simulink model "os_polyphase_synth_4096c"  (R2021a) (continued)
== system os_polyphase_synth_4096c/pfb_fir/pol1_in1_tap26 == (continued)
      | P+C+CONCAT+PCIN
      | P+C+CONCAT+PCIN+CARRYIN
      | P+C+CONCAT+PCIN>>17
      | P+C+CONCAT+PCIN>>17+CARRYIN
      | P+C+CONCAT-C
      | P+C+CONCAT-P
      | P+C+CONCAT-P>>17
      | P+C+CONCAT-PCIN
      | P+C+CONCAT-PCIN>>17
      | P+C+D
      | P+C+D*B
      | P+C+D*B+CARRYIN
      | P+C+D*BCIN
      | P+C+D*BCIN+CARRYIN
      | P+C+D*D
      | P+C+D*D+CARRYIN
      | P+C+D+CARRYIN
      | P+C+P
      | P+C+P+C
      | P+C+P+C+CARRYIN
      | P+C+P+CARRYIN
      | P+C+P+CONCAT
      | P+C+P+CONCAT+CARRYIN
      | P+C+P+P
      | P+C+P+P+CARRYIN
      | P+C+P+P>>17
      | P+C+P+P>>17+CARRYIN
      | P+C+P+PCIN
      | P+C+P+PCIN+CARRYIN
      | P+C+P+PCIN>>17
      | P+C+P+PCIN>>17+CARRYIN
      | P+C+P-C
      | P+C+P-P
      | P+C+P-P>>17
      | P+C+P-PCIN
      | P+C+P-PCIN>>17
      | P+C+P>>17
      | P+C+P>>17+C
      | P+C+P>>17+C+CARRYIN
      | P+C+P>>17+CARRYIN
      | P+C+P>>17+CONCAT
      | P+C+P>>17+CONCAT+CARRYIN
      | P+C+P>>17+P
      | P+C+P>>17+P+CARRYIN
      | P+C+PCIN
      | P+C+PCIN+C
      | P+C+PCIN+C+CARRYIN
      | P+C+PCIN+CARRYIN
      | P+C+PCIN+CONCAT
      | P+C+PCIN+CONCAT+CARRYIN
      | P+C+PCIN+P
      | P+C+PCIN+P+CARRYIN
      | P+C+PCIN>>17
      | P+C+PCIN>>17+C
      | P+C+PCIN>>17+C+CARRYIN
      | P+C+PCIN>>17+CARRYIN
      | P+C+PCIN>>17+CONCAT
      | P+C+PCIN>>17+CONCAT+CARRYIN
      | P+C+PCIN>>17+P
      | P+C+PCIN>>17+P+CARRYIN
      | P+C-C
      | P+C-P
      | P+C-P>>17
      | P+C-PCIN
      | P+C-PCIN>>17
      | P+CARRYCASCIN
      | P+CARRYIN
      | P+CONCAT
      | P+CONCAT+C
      | P+CONCAT+C+C
      | P+CONCAT+C+C+CARRYIN
      | P+CONCAT+C+CARRYIN
      | P+CONCAT+C+P
      | P+CONCAT+C+P+CARRYIN
      | P+CONCAT+C+P>>17
      | P+CONCAT+C+P>>17+CARRYIN
      | P+CONCAT+C+PCIN
      | P+CONCAT+C+PCIN+CARRYIN
      | P+CONCAT+C+PCIN>>17
      | P+CONCAT+C+PCIN>>17+CARRYIN
      | P+CONCAT+C-C
      | P+CONCAT+C-P
      | P+CONCAT+C-P>>17
      | P+CONCAT+C-PCIN
      | P+CONCAT+C-PCIN>>17
      | P+CONCAT+CARRYCASCIN
      | P+CONCAT+CARRYIN
      | P+CONCAT+P
      | P+CONCAT+P+C
      | P+CONCAT+P+C+CARRYIN
      | P+CONCAT+P+CARRYIN
      | P+CONCAT+P>>17
      | P+CONCAT+P>>17+C
      | P+CONCAT+P>>17+C+CARRYIN
      | P+CONCAT+P>>17+CARRYIN
      | P+CONCAT+PCIN
      | P+CONCAT+PCIN+C
      | P+CONCAT+PCIN+C+CARRYIN
      | P+CONCAT+PCIN+CARRYIN
      | P+CONCAT+PCIN>>17
      | P+CONCAT+PCIN>>17+C
      | P+CONCAT+PCIN>>17+C+CARRYIN
      | P+CONCAT+PCIN>>17+CARRYIN
      | P+CONCAT-C
      | P+CONCAT-P
      | P+CONCAT-P>>17
      | P+CONCAT-PCIN
      | P+CONCAT-PCIN>>17
      | P+D
      | P+D*B
      | P+D*B+C
      | P+D*B+C+CARRYIN
      | P+D*B+CARRYIN
      | P+D*B+P
      | P+D*B+P+CARRYIN
      | P+D*B+P>>17
      | P+D*B+P>>17+CARRYIN
      | P+D*B+PCIN
      | P+D*B+PCIN+CARRYIN
      | P+D*B+PCIN>>17
      | P+D*B+PCIN>>17+CARRYIN
      | P+D*B-C
      | P+D*B-P
      | P+D*B-P>>17
      | P+D*B-PCIN
      | P+D*B-PCIN>>17
      | P+D*BCIN
      | P+D*BCIN+C
      | P+D*BCIN+C+CARRYIN
      | P+D*BCIN+CARRYIN
      | P+D*BCIN+P
      | P+D*BCIN+P+CARRYIN
      | P+D*BCIN+P>>17
      | P+D*BCIN+P>>17+CARRYIN
      | P+D*BCIN+PCIN
      | P+D*BCIN+PCIN+CARRYIN
      | P+D*BCIN+PCIN>>17
      | P+D*BCIN+PCIN>>17+CARRYIN
      | P+D*BCIN-C
      | P+D*BCIN-P
      | P+D*BCIN-P>>17
      | P+D*BCIN-PCIN
      | P+D*BCIN-PCIN>>17
      | P+D*D
      | P+D*D+C
      | P+D*D+C+CARRYIN
      | P+D*D+CARRYIN
      | P+D*D+P
      | P+D*D+P+CARRYIN
      | P+D*D+P>>17
      | P+D*D+P>>17+CARRYIN
      | P+D*D+PCIN
      | P+D*D+PCIN+CARRYIN
      | P+D*D+PCIN>>17
      | P+D*D+PCIN>>17+CARRYIN
      | P+D*D-C
      | P+D*D-P
      | P+D*D-P>>17
      | P+D*D-PCIN
      | P+D*D-PCIN>>17
      | P+D+C
      | P+D+C+CARRYIN
      | P+D+CARRYIN
      | P+D+P
      | P+D+P+CARRYIN
      | P+D+P>>17
      | P+D+P>>17+CARRYIN
      | P+D+PCIN
      | P+D+PCIN+CARRYIN
      | P+D+PCIN>>17
      | P+D+PCIN>>17+CARRYIN
      | P+D-C
      | P+D-P
      | P+D-P>>17
      | P+D-PCIN
      | P+D-PCIN>>17
      | P+P
      | P+P+(A+D)
      | P+P+(A+D)*(A+D)
      | P+P+(A+D)*(A+D)+CARRYIN
      | P+P+(A+D)*B
      | P+P+(A+D)*B+CARRYIN
      | P+P+(A+D)*BCIN
      | P+P+(A+D)*BCIN+CARRYIN
      | P+P+(A+D)+CARRYIN
      | P+P+(ACIN+D)
      | P+P+(ACIN+D)*(ACIN+D)
      | P+P+(ACIN+D)*(ACIN+D)+CARRYIN
      | P+P+(ACIN+D)*B
      | P+P+(ACIN+D)*B+CARRYIN
      | P+P+(ACIN+D)*BCIN
      | P+P+(ACIN+D)*BCIN+CARRYIN
      | P+P+(ACIN+D)+CARRYIN
      | P+P+(D+A)
      | P+P+(D+A)*(D+A)
      | P+P+(D+A)*(D+A)+CARRYIN
      | P+P+(D+A)*B
      | P+P+(D+A)*B+CARRYIN
      | P+P+(D+A)*BCIN
      | P+P+(D+A)*BCIN+CARRYIN
      | P+P+(D+A)+CARRYIN
      | P+P+(D+ACIN)
      | P+P+(D+ACIN)*(D+ACIN)
      | P+P+(D+ACIN)*(D+ACIN)+CARRYIN
      | P+P+(D+ACIN)*B
      | P+P+(D+ACIN)*B+CARRYIN
      | P+P+(D+ACIN)*BCIN
      | P+P+(D+ACIN)*BCIN+CARRYIN
      | P+P+(D+ACIN)+CARRYIN
      | P+P+(D-A)
      | P+P+(D-A)*(D-A)
      | P+P+(D-A)*(D-A)+CARRYIN
      | P+P+(D-A)*B
      | P+P+(D-A)*B+CARRYIN
      | P+P+(D-A)*BCIN
      | P+P+(D-A)*BCIN+CARRYIN
      | P+P+(D-A)+CARRYIN
      | P+P+(D-ACIN)
      | P+P+(D-ACIN)*(D-ACIN)
      | P+P+(D-ACIN)*(D-ACIN)+CARRYIN
      | P+P+(D-ACIN)*B
      | P+P+(D-ACIN)*B+CARRYIN
      | P+P+(D-ACIN)*BCIN
      | P+P+(D-ACIN)*BCIN+CARRYIN
      | P+P+(D-ACIN)+CARRYIN
      | P+P+A
      | P+P+A*A
      | P+P+A*A+CARRYIN
      | P+P+A*B
      | P+P+A*B+CARRYIN
      | P+P+A*BCIN
      | P+P+A*BCIN+CARRYIN
      | P+P+A+CARRYIN
      | P+P+ACIN
      | P+P+ACIN*ACIN
      | P+P+ACIN*ACIN+CARRYIN
      | P+P+ACIN*B
      | P+P+ACIN*B+CARRYIN
      | P+P+ACIN*BCIN
      | P+P+ACIN*BCIN+CARRYIN
      | P+P+ACIN+CARRYIN
      | P+P+B*(A+D)
      | P+P+B*(A+D)+CARRYIN
      | P+P+B*(ACIN+D)
      | P+P+B*(ACIN+D)+CARRYIN
      | P+P+B*(D+A)
      | P+P+B*(D+A)+CARRYIN
      | P+P+B*(D+ACIN)
      | P+P+B*(D+ACIN)+CARRYIN
      | P+P+B*(D-A)
      | P+P+B*(D-A)+CARRYIN
      | P+P+B*(D-ACIN)
      | P+P+B*(D-ACIN)+CARRYIN
      | P+P+B*A
      | P+P+B*A+CARRYIN
      | P+P+B*ACIN
      | P+P+B*ACIN+CARRYIN
      | P+P+B*D
      | P+P+B*D+CARRYIN
      | P+P+BCIN*(A+D)
      | P+P+BCIN*(A+D)+CARRYIN
      | P+P+BCIN*(ACIN+D)
      | P+P+BCIN*(ACIN+D)+CARRYIN
      | P+P+BCIN*(D+A)
      | P+P+BCIN*(D+A)+CARRYIN
      | P+P+BCIN*(D+ACIN)
      | P+P+BCIN*(D+ACIN)+CARRYIN
      | P+P+BCIN*(D-A)
      | P+P+BCIN*(D-A)+CARRYIN
      | P+P+BCIN*(D-ACIN)
      | P+P+BCIN*(D-ACIN)+CARRYIN
      | P+P+BCIN*A
      | P+P+BCIN*A+CARRYIN
      | P+P+BCIN*ACIN
      | P+P+BCIN*ACIN+CARRYIN
      | P+P+BCIN*D
      | P+P+BCIN*D+CARRYIN
      | P+P+C
      | P+P+C+C
      | P+P+C+C+CARRYIN
      | P+P+C+CARRYIN
      | P+P+C+CONCAT
      | P+P+C+CONCAT+CARRYIN
      | P+P+C+P
      | P+P+C+P+CARRYIN
      | P+P+C+P>>17
      | P+P+C+P>>17+CARRYIN
      | P+P+C+PCIN
      | P+P+C+PCIN+CARRYIN
      | P+P+C+PCIN>>17
      | P+P+C+PCIN>>17+CARRYIN
      | P+P+C-C
      | P+P+C-P
      | P+P+C-P>>17
      | P+P+C-PCIN
      | P+P+C-PCIN>>17
      | P+P+CARRYCASCIN
      | P+P+CARRYIN
      | P+P+CONCAT
      | P+P+CONCAT+C
      | P+P+CONCAT+C+CARRYIN
      | P+P+CONCAT+CARRYIN
      | P+P+D
      | P+P+D*B
      | P+P+D*B+CARRYIN
      | P+P+D*BCIN
      | P+P+D*BCIN+CARRYIN
      | P+P+D*D
      | P+P+D*D+CARRYIN
      | P+P+D+CARRYIN
      | P+P+P
      | P+P+P+C
      | P+P+P+C+CARRYIN
      | P+P+P+CARRYIN
      | P+P+P>>17
      | P+P+P>>17+C
      | P+P+P>>17+C+CARRYIN
      | P+P+P>>17+CARRYIN
      | P+P+PCIN
      | P+P+PCIN+C
      | P+P+PCIN+C+CARRYIN
      | P+P+PCIN+CARRYIN
      | P+P+PCIN>>17
      | P+P+PCIN>>17+C
      | P+P+PCIN>>17+C+CARRYIN
      | P+P+PCIN>>17+CARRYIN
      | P+P-C
      | P+P-P
      | P+P-P>>17
      | P+P-PCIN
      | P+P-PCIN>>17
      | P+P>>17
      | P+P>>17+(A+D)
      | P+P>>17+(A+D)*(A+D)
      | P+P>>17+(A+D)*(A+D)+CARRYIN
      | P+P>>17+(A+D)*B
      | P+P>>17+(A+D)*B+CARRYIN
      | P+P>>17+(A+D)*BCIN
      | P+P>>17+(A+D)*BCIN+CARRYIN
      | P+P>>17+(A+D)+CARRYIN
      | P+P>>17+(ACIN+D)
      | P+P>>17+(ACIN+D)*(ACIN+D)
      | P+P>>17+(ACIN+D)*(ACIN+D)+CARRYIN
      | P+P>>17+(ACIN+D)*B
      | P+P>>17+(ACIN+D)*B+CARRYIN
      | P+P>>17+(ACIN+D)*BCIN
      | P+P>>17+(ACIN+D)*BCIN+CARRYIN
      | P+P>>17+(ACIN+D)+CARRYIN
      | P+P>>17+(D+A)
      | P+P>>17+(D+A)*(D+A)
      | P+P>>17+(D+A)*(D+A)+CARRYIN
      | P+P>>17+(D+A)*B
      | P+P>>17+(D+A)*B+CARRYIN
      | P+P>>17+(D+A)*BCIN
      | P+P>>17+(D+A)*BCIN+CARRYIN
      | P+P>>17+(D+A)+CARRYIN
      | P+P>>17+(D+ACIN)
      | P+P>>17+(D+ACIN)*(D+ACIN)
      | P+P>>17+(D+ACIN)*(D+ACIN)+CARRYIN
      | P+P>>17+(D+ACIN)*B
      | P+P>>17+(D+ACIN)*B+CARRYIN
      | P+P>>17+(D+ACIN)*BCIN
      | P+P>>17+(D+ACIN)*BCIN+CARRYIN
      | P+P>>17+(D+ACIN)+CARRYIN
      | P+P>>17+(D-A)
      | P+P>>17+(D-A)*(D-A)
      | P+P>>17+(D-A)*(D-A)+CARRYIN
      | P+P>>17+(D-A)*B
      | P+P>>17+(D-A)*B+CARRYIN
      | P+P>>17+(D-A)*BCIN
      | P+P>>17+(D-A)*BCIN+CARRYIN
      | P+P>>17+(D-A)+CARRYIN
      | P+P>>17+(D-ACIN)
      | P+P>>17+(D-ACIN)*(D-ACIN)
      | P+P>>17+(D-ACIN)*(D-ACIN)+CARRYIN
      | P+P>>17+(D-ACIN)*B
      | P+P>>17+(D-ACIN)*B+CARRYIN
      | P+P>>17+(D-ACIN)*BCIN
      | P+P>>17+(D-ACIN)*BCIN+CARRYIN
      | P+P>>17+(D-ACIN)+CARRYIN
      | P+P>>17+A
      | P+P>>17+A*A
      | P+P>>17+A*A+CARRYIN
      | P+P>>17+A*B
      | P+P>>17+A*B+CARRYIN
      | P+P>>17+A*BCIN
      | P+P>>17+A*BCIN+CARRYIN
      | P+P>>17+A+CARRYIN
      | P+P>>17+ACIN
      | P+P>>17+ACIN*ACIN
      | P+P>>17+ACIN*ACIN+CARRYIN
      | P+P>>17+ACIN*B
      | P+P>>17+ACIN*B+CARRYIN
      | P+P>>17+ACIN*BCIN
      | P+P>>17+ACIN*BCIN+CARRYIN
      | P+P>>17+ACIN+CARRYIN
      | P+P>>17+B*(A+D)
      | P+P>>17+B*(A+D)+CARRYIN
      | P+P>>17+B*(ACIN+D)
      | P+P>>17+B*(ACIN+D)+CARRYIN
      | P+P>>17+B*(D+A)
      | P+P>>17+B*(D+A)+CARRYIN
      | P+P>>17+B*(D+ACIN)
      | P+P>>17+B*(D+ACIN)+CARRYIN
      | P+P>>17+B*(D-A)
      | P+P>>17+B*(D-A)+CARRYIN
      | P+P>>17+B*(D-ACIN)
      | P+P>>17+B*(D-ACIN)+CARRYIN
      | P+P>>17+B*A
      | P+P>>17+B*A+CARRYIN
      | P+P>>17+B*ACIN
      | P+P>>17+B*ACIN+CARRYIN
      | P+P>>17+B*D
      | P+P>>17+B*D+CARRYIN
      | P+P>>17+BCIN*(A+D)
      | P+P>>17+BCIN*(A+D)+CARRYIN
      | P+P>>17+BCIN*(ACIN+D)
      | P+P>>17+BCIN*(ACIN+D)+CARRYIN
      | P+P>>17+BCIN*(D+A)
      | P+P>>17+BCIN*(D+A)+CARRYIN
      | P+P>>17+BCIN*(D+ACIN)
      | P+P>>17+BCIN*(D+ACIN)+CARRYIN
      | P+P>>17+BCIN*(D-A)
      | P+P>>17+BCIN*(D-A)+CARRYIN
      | P+P>>17+BCIN*(D-ACIN)
      | P+P>>17+BCIN*(D-ACIN)+CARRYIN
      | P+P>>17+BCIN*A
      | P+P>>17+BCIN*A+CARRYIN
      | P+P>>17+BCIN*ACIN
      | P+P>>17+BCIN*ACIN+CARRYIN
      | P+P>>17+BCIN*D
      | P+P>>17+BCIN*D+CARRYIN
      | P+P>>17+C
      | P+P>>17+C+C
      | P+P>>17+C+C+CARRYIN
      | P+P>>17+C+CARRYIN
      | P+P>>17+C+CONCAT
      | P+P>>17+C+CONCAT+CARRYIN
      | P+P>>17+C+P
      | P+P>>17+C+P+CARRYIN
      | P+P>>17+CARRYIN
      | P+P>>17+CONCAT
      | P+P>>17+CONCAT+C
      | P+P>>17+CONCAT+C+CARRYIN
      | P+P>>17+CONCAT+CARRYIN
      | P+P>>17+D
      | P+P>>17+D*B
      | P+P>>17+D*B+CARRYIN
      | P+P>>17+D*BCIN
      | P+P>>17+D*BCIN+CARRYIN
      | P+P>>17+D*D
      | P+P>>17+D*D+CARRYIN
      | P+P>>17+D+CARRYIN
      | P+P>>17+P
      | P+P>>17+P+C
      | P+P>>17+P+C+CARRYIN
      | P+P>>17+P+CARRYIN
      | P+PCIN
      | P+PCIN+(A+D)
      | P+PCIN+(A+D)*(A+D)
      | P+PCIN+(A+D)*(A+D)+CARRYIN
      | P+PCIN+(A+D)*B
      | P+PCIN+(A+D)*B+CARRYIN
      | P+PCIN+(A+D)*BCIN
      | P+PCIN+(A+D)*BCIN+CARRYIN
      | P+PCIN+(A+D)+CARRYIN
      | P+PCIN+(ACIN+D)
      | P+PCIN+(ACIN+D)*(ACIN+D)
      | P+PCIN+(ACIN+D)*(ACIN+D)+CARRYIN
      | P+PCIN+(ACIN+D)*B
      | P+PCIN+(ACIN+D)*B+CARRYIN
      | P+PCIN+(ACIN+D)*BCIN
      | P+PCIN+(ACIN+D)*BCIN+CARRYIN
      | P+PCIN+(ACIN+D)+CARRYIN
      | P+PCIN+(D+A)
      | P+PCIN+(D+A)*(D+A)
      | P+PCIN+(D+A)*(D+A)+CARRYIN
      | P+PCIN+(D+A)*B
      | P+PCIN+(D+A)*B+CARRYIN
      | P+PCIN+(D+A)*BCIN
      | P+PCIN+(D+A)*BCIN+CARRYIN
      | P+PCIN+(D+A)+CARRYIN
      | P+PCIN+(D+ACIN)
      | P+PCIN+(D+ACIN)*(D+ACIN)
      | P+PCIN+(D+ACIN)*(D+ACIN)+CARRYIN
      | P+PCIN+(D+ACIN)*B
      | P+PCIN+(D+ACIN)*B+CARRYIN
      | P+PCIN+(D+ACIN)*BCIN
      | P+PCIN+(D+ACIN)*BCIN+CARRYIN
      | P+PCIN+(D+ACIN)+CARRYIN
      | P+PCIN+(D-A)
      | P+PCIN+(D-A)*(D-A)
      | P+PCIN+(D-A)*(D-A)+CARRYIN
      | P+PCIN+(D-A)*B
      | P+PCIN+(D-A)*B+CARRYIN
      | P+PCIN+(D-A)*BCIN
      | P+PCIN+(D-A)*BCIN+CARRYIN
      | P+PCIN+(D-A)+CARRYIN
      | P+PCIN+(D-ACIN)
      | P+PCIN+(D-ACIN)*(D-ACIN)
      | P+PCIN+(D-ACIN)*(D-ACIN)+CARRYIN
      | P+PCIN+(D-ACIN)*B
      | P+PCIN+(D-ACIN)*B+CARRYIN
      | P+PCIN+(D-ACIN)*BCIN
      | P+PCIN+(D-ACIN)*BCIN+CARRYIN
      | P+PCIN+(D-ACIN)+CARRYIN
      | P+PCIN+A
      | P+PCIN+A*A
      | P+PCIN+A*A+CARRYIN
      | P+PCIN+A*B
      | P+PCIN+A*B+CARRYIN
      | P+PCIN+A*BCIN
      | P+PCIN+A*BCIN+CARRYIN
      | P+PCIN+A+CARRYIN
      | P+PCIN+ACIN
      | P+PCIN+ACIN*ACIN
      | P+PCIN+ACIN*ACIN+CARRYIN
      | P+PCIN+ACIN*B
      | P+PCIN+ACIN*B+CARRYIN
      | P+PCIN+ACIN*BCIN
      | P+PCIN+ACIN*BCIN+CARRYIN
      | P+PCIN+ACIN+CARRYIN
      | P+PCIN+B*(A+D)
      | P+PCIN+B*(A+D)+CARRYIN
      | P+PCIN+B*(ACIN+D)
      | P+PCIN+B*(ACIN+D)+CARRYIN
      | P+PCIN+B*(D+A)
      | P+PCIN+B*(D+A)+CARRYIN
      | P+PCIN+B*(D+ACIN)
      | P+PCIN+B*(D+ACIN)+CARRYIN
      | P+PCIN+B*(D-A)
      | P+PCIN+B*(D-A)+CARRYIN
      | P+PCIN+B*(D-ACIN)
      | P+PCIN+B*(D-ACIN)+CARRYIN
      | P+PCIN+B*A
      | P+PCIN+B*A+CARRYIN
      | P+PCIN+B*ACIN
      | P+PCIN+B*ACIN+CARRYIN
      | P+PCIN+B*D
      | P+PCIN+B*D+CARRYIN
      | P+PCIN+BCIN*(A+D)
      | P+PCIN+BCIN*(A+D)+CARRYIN
      | P+PCIN+BCIN*(ACIN+D)
      | P+PCIN+BCIN*(ACIN+D)+CARRYIN
      | P+PCIN+BCIN*(D+A)
      | P+PCIN+BCIN*(D+A)+CARRYIN
      | P+PCIN+BCIN*(D+ACIN)
      | P+PCIN+BCIN*(D+ACIN)+CARRYIN
      | P+PCIN+BCIN*(D-A)
      | P+PCIN+BCIN*(D-A)+CARRYIN
      | P+PCIN+BCIN*(D-ACIN)
      | P+PCIN+BCIN*(D-ACIN)+CARRYIN
      | P+PCIN+BCIN*A
      | P+PCIN+BCIN*A+CARRYIN
      | P+PCIN+BCIN*ACIN
      | P+PCIN+BCIN*ACIN+CARRYIN
      | P+PCIN+BCIN*D
      | P+PCIN+BCIN*D+CARRYIN
      | P+PCIN+C
      | P+PCIN+C+C
      | P+PCIN+C+C+CARRYIN
      | P+PCIN+C+CARRYIN
      | P+PCIN+C+CONCAT
      | P+PCIN+C+CONCAT+CARRYIN
      | P+PCIN+C+P
      | P+PCIN+C+P+CARRYIN
      | P+PCIN+CARRYIN
      | P+PCIN+CONCAT
      | P+PCIN+CONCAT+C
      | P+PCIN+CONCAT+C+CARRYIN
      | P+PCIN+CONCAT+CARRYIN
      | P+PCIN+D
      | P+PCIN+D*B
      | P+PCIN+D*B+CARRYIN
      | P+PCIN+D*BCIN
      | P+PCIN+D*BCIN+CARRYIN
      | P+PCIN+D*D
      | P+PCIN+D*D+CARRYIN
      | P+PCIN+D+CARRYIN
      | P+PCIN+P
      | P+PCIN+P+C
      | P+PCIN+P+C+CARRYIN
      | P+PCIN+P+CARRYIN
      | P+PCIN>>17
      | P+PCIN>>17+(A+D)
      | P+PCIN>>17+(A+D)*(A+D)
      | P+PCIN>>17+(A+D)*(A+D)+CARRYIN
      | P+PCIN>>17+(A+D)*B
      | P+PCIN>>17+(A+D)*B+CARRYIN
      | P+PCIN>>17+(A+D)*BCIN
      | P+PCIN>>17+(A+D)*BCIN+CARRYIN
      | P+PCIN>>17+(A+D)+CARRYIN
      | P+PCIN>>17+(ACIN+D)
      | P+PCIN>>17+(ACIN+D)*(ACIN+D)
      | P+PCIN>>17+(ACIN+D)*(ACIN+D)+CARRYIN
      | P+PCIN>>17+(ACIN+D)*B
      | P+PCIN>>17+(ACIN+D)*B+CARRYIN
      | P+PCIN>>17+(ACIN+D)*BCIN
      | P+PCIN>>17+(ACIN+D)*BCIN+CARRYIN
      | P+PCIN>>17+(ACIN+D)+CARRYIN
      | P+PCIN>>17+(D+A)
      | P+PCIN>>17+(D+A)*(D+A)
      | P+PCIN>>17+(D+A)*(D+A)+CARRYIN
      | P+PCIN>>17+(D+A)*B
      | P+PCIN>>17+(D+A)*B+CARRYIN
      | P+PCIN>>17+(D+A)*BCIN
      | P+PCIN>>17+(D+A)*BCIN+CARRYIN
      | P+PCIN>>17+(D+A)+CARRYIN
      | P+PCIN>>17+(D+ACIN)
      | P+PCIN>>17+(D+ACIN)*(D+ACIN)
      | P+PCIN>>17+(D+ACIN)*(D+ACIN)+CARRYIN
      | P+PCIN>>17+(D+ACIN)*B
      | P+PCIN>>17+(D+ACIN)*B+CARRYIN
      | P+PCIN>>17+(D+ACIN)*BCIN
      | P+PCIN>>17+(D+ACIN)*BCIN+CARRYIN
      | P+PCIN>>17+(D+ACIN)+CARRYIN
      | P+PCIN>>17+(D-A)
      | P+PCIN>>17+(D-A)*(D-A)
      | P+PCIN>>17+(D-A)*(D-A)+CARRYIN
      | P+PCIN>>17+(D-A)*B
      | P+PCIN>>17+(D-A)*B+CARRYIN
      | P+PCIN>>17+(D-A)*BCIN
      | P+PCIN>>17+(D-A)*BCIN+CARRYIN
      | P+PCIN>>17+(D-A)+CARRYIN
      | P+PCIN>>17+(D-ACIN)
      | P+PCIN>>17+(D-ACIN)*(D-ACIN)
      | P+PCIN>>17+(D-ACIN)*(D-ACIN)+CARRYIN
      | P+PCIN>>17+(D-ACIN)*B
      | P+PCIN>>17+(D-ACIN)*B+CARRYIN
      | P+PCIN>>17+(D-ACIN)*BCIN
      | P+PCIN>>17+(D-ACIN)*BCIN+CARRYIN
      | P+PCIN>>17+(D-ACIN)+CARRYIN
      | P+PCIN>>17+A
      | P+PCIN>>17+A*A
      | P+PCIN>>17+A*A+CARRYIN
      | P+PCIN>>17+A*B
      | P+PCIN>>17+A*B+CARRYIN
      | P+PCIN>>17+A*BCIN
      | P+PCIN>>17+A*BCIN+CARRYIN
      | P+PCIN>>17+A+CARRYIN
      | P+PCIN>>17+ACIN
      | P+PCIN>>17+ACIN*ACIN
      | P+PCIN>>17+ACIN*ACIN+CARRYIN
      | P+PCIN>>17+ACIN*B
      | P+PCIN>>17+ACIN*B+CARRYIN
      | P+PCIN>>17+ACIN*BCIN
      | P+PCIN>>17+ACIN*BCIN+CARRYIN
      | P+PCIN>>17+ACIN+CARRYIN
      | P+PCIN>>17+B*(A+D)
      | P+PCIN>>17+B*(A+D)+CARRYIN
      | P+PCIN>>17+B*(ACIN+D)
      | P+PCIN>>17+B*(ACIN+D)+CARRYIN
      | P+PCIN>>17+B*(D+A)
      | P+PCIN>>17+B*(D+A)+CARRYIN
      | P+PCIN>>17+B*(D+ACIN)
      | P+PCIN>>17+B*(D+ACIN)+CARRYIN
      | P+PCIN>>17+B*(D-A)
      | P+PCIN>>17+B*(D-A)+CARRYIN
      | P+PCIN>>17+B*(D-ACIN)
      | P+PCIN>>17+B*(D-ACIN)+CARRYIN
      | P+PCIN>>17+B*A
      | P+PCIN>>17+B*A+CARRYIN
      | P+PCIN>>17+B*ACIN
      | P+PCIN>>17+B*ACIN+CARRYIN
      | P+PCIN>>17+B*D
      | P+PCIN>>17+B*D+CARRYIN
      | P+PCIN>>17+BCIN*(A+D)
      | P+PCIN>>17+BCIN*(A+D)+CARRYIN
      | P+PCIN>>17+BCIN*(ACIN+D)
      | P+PCIN>>17+BCIN*(ACIN+D)+CARRYIN
      | P+PCIN>>17+BCIN*(D+A)
      | P+PCIN>>17+BCIN*(D+A)+CARRYIN
      | P+PCIN>>17+BCIN*(D+ACIN)
      | P+PCIN>>17+BCIN*(D+ACIN)+CARRYIN
      | P+PCIN>>17+BCIN*(D-A)
      | P+PCIN>>17+BCIN*(D-A)+CARRYIN
      | P+PCIN>>17+BCIN*(D-ACIN)
      | P+PCIN>>17+BCIN*(D-ACIN)+CARRYIN
      | P+PCIN>>17+BCIN*A
      | P+PCIN>>17+BCIN*A+CARRYIN
      | P+PCIN>>17+BCIN*ACIN
      | P+PCIN>>17+BCIN*ACIN+CARRYIN
      | P+PCIN>>17+BCIN*D
      | P+PCIN>>17+BCIN*D+CARRYIN
      | P+PCIN>>17+C
      | P+PCIN>>17+C+C
      | P+PCIN>>17+C+C+CARRYIN
      | P+PCIN>>17+C+CARRYIN
      | P+PCIN>>17+C+CONCAT
      | P+PCIN>>17+C+CONCAT+CARRYIN
      | P+PCIN>>17+C+P
      | P+PCIN>>17+C+P+CARRYIN
      | P+PCIN>>17+CARRYIN
      | P+PCIN>>17+CONCAT
      | P+PCIN>>17+CONCAT+C
      | P+PCIN>>17+CONCAT+C+CARRYIN
      | P+PCIN>>17+CONCAT+CARRYIN
      | P+PCIN>>17+D
      | P+PCIN>>17+D*B
      | P+PCIN>>17+D*B+CARRYIN
      | P+PCIN>>17+D*BCIN
      | P+PCIN>>17+D*BCIN+CARRYIN
      | P+PCIN>>17+D*D
      | P+PCIN>>17+D*D+CARRYIN
      | P+PCIN>>17+D+CARRYIN
      | P+PCIN>>17+P
      | P+PCIN>>17+P+C
      | P+PCIN>>17+P+C+CARRYIN
      | P+PCIN>>17+P+CARRYIN
      | P-((A+D))
      | P-((A+D)*(A+D))
      | P-((A+D)*(A+D)+C)
      | P-((A+D)*(A+D)+C+CARRYIN)
      | P-((A+D)*(A+D)+CARRYIN)
      | P-((A+D)*(A+D)+P)
      | P-((A+D)*(A+D)+P+CARRYIN)
      | P-((A+D)*B)
      | P-((A+D)*B+C)
      | P-((A+D)*B+C+CARRYIN)
      | P-((A+D)*B+CARRYIN)
      | P-((A+D)*B+P)
      | P-((A+D)*B+P+CARRYIN)
      | P-((A+D)*BCIN)
      | P-((A+D)*BCIN+C)
      | P-((A+D)*BCIN+C+CARRYIN)
      | P-((A+D)*BCIN+CARRYIN)
      | P-((A+D)*BCIN+P)
      | P-((A+D)*BCIN+P+CARRYIN)
      | P-((A+D)+C)
      | P-((A+D)+C+CARRYIN)
      | P-((A+D)+CARRYIN)
      | P-((A+D)+P)
      | P-((A+D)+P+CARRYIN)
      | P-((ACIN+D))
      | P-((ACIN+D)*(ACIN+D))
      | P-((ACIN+D)*(ACIN+D)+C)
      | P-((ACIN+D)*(ACIN+D)+C+CARRYIN)
      | P-((ACIN+D)*(ACIN+D)+CARRYIN)
      | P-((ACIN+D)*(ACIN+D)+P)
      | P-((ACIN+D)*(ACIN+D)+P+CARRYIN)
      | P-((ACIN+D)*B)
      | P-((ACIN+D)*B+C)
      | P-((ACIN+D)*B+C+CARRYIN)
      | P-((ACIN+D)*B+CARRYIN)
      | P-((ACIN+D)*B+P)
      | P-((ACIN+D)*B+P+CARRYIN)
      | P-((ACIN+D)*BCIN)
      | P-((ACIN+D)*BCIN+C)
      | P-((ACIN+D)*BCIN+C+CARRYIN)
      | P-((ACIN+D)*BCIN+CARRYIN)
      | P-((ACIN+D)*BCIN+P)
      | P-((ACIN+D)*BCIN+P+CARRYIN)
      | P-((ACIN+D)+C)
      | P-((ACIN+D)+C+CARRYIN)
      | P-((ACIN+D)+CARRYIN)
      | P-((ACIN+D)+P)
      | P-((ACIN+D)+P+CARRYIN)
      | P-((D+A))
      | P-((D+A)*(D+A))
      | P-((D+A)*(D+A)+C)
      | P-((D+A)*(D+A)+C+CARRYIN)
      | P-((D+A)*(D+A)+CARRYIN)
      | P-((D+A)*(D+A)+P)
      | P-((D+A)*(D+A)+P+CARRYIN)
      | P-((D+A)*B)
      | P-((D+A)*B+C)
      | P-((D+A)*B+C+CARRYIN)
      | P-((D+A)*B+CARRYIN)
      | P-((D+A)*B+P)
      | P-((D+A)*B+P+CARRYIN)
      | P-((D+A)*BCIN)
      | P-((D+A)*BCIN+C)
      | P-((D+A)*BCIN+C+CARRYIN)
      | P-((D+A)*BCIN+CARRYIN)
      | P-((D+A)*BCIN+P)
      | P-((D+A)*BCIN+P+CARRYIN)
      | P-((D+A)+C)
      | P-((D+A)+C+CARRYIN)
      | P-((D+A)+CARRYIN)
      | P-((D+A)+P)
      | P-((D+A)+P+CARRYIN)
      | P-((D+ACIN))
      | P-((D+ACIN)*(D+ACIN))
      | P-((D+ACIN)*(D+ACIN)+C)
      | P-((D+ACIN)*(D+ACIN)+C+CARRYIN)
      | P-((D+ACIN)*(D+ACIN)+CARRYIN)
      | P-((D+ACIN)*(D+ACIN)+P)
      | P-((D+ACIN)*(D+ACIN)+P+CARRYIN)
      | P-((D+ACIN)*B)
      | P-((D+ACIN)*B+C)
      | P-((D+ACIN)*B+C+CARRYIN)
      | P-((D+ACIN)*B+CARRYIN)
      | P-((D+ACIN)*B+P)
      | P-((D+ACIN)*B+P+CARRYIN)
      | P-((D+ACIN)*BCIN)
      | P-((D+ACIN)*BCIN+C)
      | P-((D+ACIN)*BCIN+C+CARRYIN)
      | P-((D+ACIN)*BCIN+CARRYIN)
      | P-((D+ACIN)*BCIN+P)
      | P-((D+ACIN)*BCIN+P+CARRYIN)
      | P-((D+ACIN)+C)
      | P-((D+ACIN)+C+CARRYIN)
      | P-((D+ACIN)+CARRYIN)
      | P-((D+ACIN)+P)
      | P-((D+ACIN)+P+CARRYIN)
      | P-((D-A))
      | P-((D-A)*(D-A))
      | P-((D-A)*(D-A)+C)
      | P-((D-A)*(D-A)+C+CARRYIN)
      | P-((D-A)*(D-A)+CARRYIN)
      | P-((D-A)*(D-A)+P)
      | P-((D-A)*(D-A)+P+CARRYIN)
      | P-((D-A)*B)
      | P-((D-A)*B+C)
      | P-((D-A)*B+C+CARRYIN)
      | P-((D-A)*B+CARRYIN)
      | P-((D-A)*B+P)
      | P-((D-A)*B+P+CARRYIN)
      | P-((D-A)*BCIN)
      | P-((D-A)*BCIN+C)
      | P-((D-A)*BCIN+C+CARRYIN)
      | P-((D-A)*BCIN+CARRYIN)
      | P-((D-A)*BCIN+P)
      | P-((D-A)*BCIN+P+CARRYIN)
      | P-((D-A)+C)
      | P-((D-A)+C+CARRYIN)
      | P-((D-A)+CARRYIN)
      | P-((D-A)+P)
      | P-((D-A)+P+CARRYIN)
      | P-((D-ACIN))
      | P-((D-ACIN)*(D-ACIN))
      | P-((D-ACIN)*(D-ACIN)+C)
      | P-((D-ACIN)*(D-ACIN)+C+CARRYIN)
      | P-((D-ACIN)*(D-ACIN)+CARRYIN)
      | P-((D-ACIN)*(D-ACIN)+P)
      | P-((D-ACIN)*(D-ACIN)+P+CARRYIN)
      | P-((D-ACIN)*B)
      | P-((D-ACIN)*B+C)
      | P-((D-ACIN)*B+C+CARRYIN)
      | P-((D-ACIN)*B+CARRYIN)
      | P-((D-ACIN)*B+P)
      | P-((D-ACIN)*B+P+CARRYIN)
      | P-((D-ACIN)*BCIN)
      | P-((D-ACIN)*BCIN+C)
      | P-((D-ACIN)*BCIN+C+CARRYIN)
      | P-((D-ACIN)*BCIN+CARRYIN)
      | P-((D-ACIN)*BCIN+P)
      | P-((D-ACIN)*BCIN+P+CARRYIN)
      | P-((D-ACIN)+C)
      | P-((D-ACIN)+C+CARRYIN)
      | P-((D-ACIN)+CARRYIN)
      | P-((D-ACIN)+P)
      | P-((D-ACIN)+P+CARRYIN)
      | P-(A)
      | P-(A*A)
      | P-(A*A+C)
      | P-(A*A+C+CARRYIN)
      | P-(A*A+CARRYIN)
      | P-(A*A+P)
      | P-(A*A+P+CARRYIN)
      | P-(A*B)
      | P-(A*B+C)
      | P-(A*B+C+CARRYIN)
      | P-(A*B+CARRYIN)
      | P-(A*B+P)
      | P-(A*B+P+CARRYIN)
      | P-(A*BCIN)
      | P-(A*BCIN+C)
      | P-(A*BCIN+C+CARRYIN)
      | P-(A*BCIN+CARRYIN)
      | P-(A*BCIN+P)
      | P-(A*BCIN+P+CARRYIN)
      | P-(A+C)
      | P-(A+C+CARRYIN)
      | P-(A+CARRYIN)
      | P-(A+D)
      | P-(A+D)*(A+D)
      | P-(A+D)*(A+D)-C
      | P-(A+D)*(A+D)-C-CARRYIN
      | P-(A+D)*(A+D)-CARRYIN
      | P-(A+D)*(A+D)-P
      | P-(A+D)*(A+D)-P-CARRYIN
      | P-(A+D)*B
      | P-(A+D)*B-C
      | P-(A+D)*B-C-CARRYIN
      | P-(A+D)*B-CARRYIN
      | P-(A+D)*B-P
      | P-(A+D)*B-P-CARRYIN
      | P-(A+D)*BCIN
      | P-(A+D)*BCIN-C
      | P-(A+D)*BCIN-C-CARRYIN
      | P-(A+D)*BCIN-CARRYIN
      | P-(A+D)*BCIN-P
      | P-(A+D)*BCIN-P-CARRYIN
      | P-(A+D)-C
      | P-(A+D)-C-CARRYIN
      | P-(A+D)-CARRYIN
      | P-(A+D)-P
      | P-(A+D)-P-CARRYIN
      | P-(A+P)
      | P-(A+P+CARRYIN)
      | P-(ACIN)
      | P-(ACIN*ACIN)
      | P-(ACIN*ACIN+C)
      | P-(ACIN*ACIN+C+CARRYIN)
      | P-(ACIN*ACIN+CARRYIN)
      | P-(ACIN*ACIN+P)
      | P-(ACIN*ACIN+P+CARRYIN)
      | P-(ACIN*B)
      | P-(ACIN*B+C)
      | P-(ACIN*B+C+CARRYIN)
      | P-(ACIN*B+CARRYIN)
      | P-(ACIN*B+P)
      | P-(ACIN*B+P+CARRYIN)
      | P-(ACIN*BCIN)
      | P-(ACIN*BCIN+C)
      | P-(ACIN*BCIN+C+CARRYIN)
      | P-(ACIN*BCIN+CARRYIN)
      | P-(ACIN*BCIN+P)
      | P-(ACIN*BCIN+P+CARRYIN)
      | P-(ACIN+C)
      | P-(ACIN+C+CARRYIN)
      | P-(ACIN+CARRYIN)
      | P-(ACIN+D)
      | P-(ACIN+D)*(ACIN+D)
      | P-(ACIN+D)*(ACIN+D)-C
      | P-(ACIN+D)*(ACIN+D)-C-CARRYIN
      | P-(ACIN+D)*(ACIN+D)-CARRYIN
      | P-(ACIN+D)*(ACIN+D)-P
      | P-(ACIN+D)*(ACIN+D)-P-CARRYIN
      | P-(ACIN+D)*B
      | P-(ACIN+D)*B-C
      | P-(ACIN+D)*B-C-CARRYIN
      | P-(ACIN+D)*B-CARRYIN
      | P-(ACIN+D)*B-P
      | P-(ACIN+D)*B-P-CARRYIN
      | P-(ACIN+D)*BCIN
      | P-(ACIN+D)*BCIN-C
      | P-(ACIN+D)*BCIN-C-CARRYIN
      | P-(ACIN+D)*BCIN-CARRYIN
      | P-(ACIN+D)*BCIN-P
      | P-(ACIN+D)*BCIN-P-CARRYIN
      | P-(ACIN+D)-C
      | P-(ACIN+D)-C-CARRYIN
      | P-(ACIN+D)-CARRYIN
      | P-(ACIN+D)-P
      | P-(ACIN+D)-P-CARRYIN
      | P-(ACIN+P)
      | P-(ACIN+P+CARRYIN)
      | P-(B*(A+D))
      | P-(B*(A+D)+C)
      | P-(B*(A+D)+C+CARRYIN)
      | P-(B*(A+D)+CARRYIN)
      | P-(B*(A+D)+P)
      | P-(B*(A+D)+P+CARRYIN)
      | P-(B*(ACIN+D))
      | P-(B*(ACIN+D)+C)
      | P-(B*(ACIN+D)+C+CARRYIN)
      | P-(B*(ACIN+D)+CARRYIN)
      | P-(B*(ACIN+D)+P)
      | P-(B*(ACIN+D)+P+CARRYIN)
      | P-(B*(D+A))
      | P-(B*(D+A)+C)
      | P-(B*(D+A)+C+CARRYIN)
      | P-(B*(D+A)+CARRYIN)
      | P-(B*(D+A)+P)
      | P-(B*(D+A)+P+CARRYIN)
      | P-(B*(D+ACIN))
      | P-(B*(D+ACIN)+C)
      | P-(B*(D+ACIN)+C+CARRYIN)
      | P-(B*(D+ACIN)+CARRYIN)
      | P-(B*(D+ACIN)+P)
      | P-(B*(D+ACIN)+P+CARRYIN)
      | P-(B*(D-A))
      | P-(B*(D-A)+C)
      | P-(B*(D-A)+C+CARRYIN)
      | P-(B*(D-A)+CARRYIN)
      | P-(B*(D-A)+P)
      | P-(B*(D-A)+P+CARRYIN)
      | P-(B*(D-ACIN))
      | P-(B*(D-ACIN)+C)
      | P-(B*(D-ACIN)+C+CARRYIN)
      | P-(B*(D-ACIN)+CARRYIN)
      | P-(B*(D-ACIN)+P)
      | P-(B*(D-ACIN)+P+CARRYIN)
      | P-(B*A)
      | P-(B*A+C)
      | P-(B*A+C+CARRYIN)
      | P-(B*A+CARRYIN)
      | P-(B*A+P)
      | P-(B*A+P+CARRYIN)
      | P-(B*ACIN)
      | P-(B*ACIN+C)
      | P-(B*ACIN+C+CARRYIN)
      | P-(B*ACIN+CARRYIN)
      | P-(B*ACIN+P)
      | P-(B*ACIN+P+CARRYIN)
      | P-(B*D)
      | P-(B*D+C)
      | P-(B*D+C+CARRYIN)
      | P-(B*D+CARRYIN)
      | P-(B*D+P)
      | P-(B*D+P+CARRYIN)
      | P-(BCIN*(A+D))
      | P-(BCIN*(A+D)+C)
      | P-(BCIN*(A+D)+C+CARRYIN)
      | P-(BCIN*(A+D)+CARRYIN)
      | P-(BCIN*(A+D)+P)
      | P-(BCIN*(A+D)+P+CARRYIN)
      | P-(BCIN*(ACIN+D))
      | P-(BCIN*(ACIN+D)+C)
      | P-(BCIN*(ACIN+D)+C+CARRYIN)
      | P-(BCIN*(ACIN+D)+CARRYIN)
      | P-(BCIN*(ACIN+D)+P)
      | P-(BCIN*(ACIN+D)+P+CARRYIN)
      | P-(BCIN*(D+A))
      | P-(BCIN*(D+A)+C)
      | P-(BCIN*(D+A)+C+CARRYIN)
      | P-(BCIN*(D+A)+CARRYIN)
      | P-(BCIN*(D+A)+P)
      | P-(BCIN*(D+A)+P+CARRYIN)
      | P-(BCIN*(D+ACIN))
      | P-(BCIN*(D+ACIN)+C)
      | P-(BCIN*(D+ACIN)+C+CARRYIN)
      | P-(BCIN*(D+ACIN)+CARRYIN)
      | P-(BCIN*(D+ACIN)+P)
      | P-(BCIN*(D+ACIN)+P+CARRYIN)
      | P-(BCIN*(D-A))
      | P-(BCIN*(D-A)+C)
      | P-(BCIN*(D-A)+C+CARRYIN)
      | P-(BCIN*(D-A)+CARRYIN)
      | P-(BCIN*(D-A)+P)
      | P-(BCIN*(D-A)+P+CARRYIN)
      | P-(BCIN*(D-ACIN))
      | P-(BCIN*(D-ACIN)+C)
      | P-(BCIN*(D-ACIN)+C+CARRYIN)
      | P-(BCIN*(D-ACIN)+CARRYIN)
      | P-(BCIN*(D-ACIN)+P)
      | P-(BCIN*(D-ACIN)+P+CARRYIN)
      | P-(BCIN*A)
      | P-(BCIN*A+C)
      | P-(BCIN*A+C+CARRYIN)
      | P-(BCIN*A+CARRYIN)
      | P-(BCIN*A+P)
      | P-(BCIN*A+P+CARRYIN)
      | P-(BCIN*ACIN)
      | P-(BCIN*ACIN+C)
      | P-(BCIN*ACIN+C+CARRYIN)
      | P-(BCIN*ACIN+CARRYIN)
      | P-(BCIN*ACIN+P)
      | P-(BCIN*ACIN+P+CARRYIN)
      | P-(BCIN*D)
      | P-(BCIN*D+C)
      | P-(BCIN*D+C+CARRYIN)
      | P-(BCIN*D+CARRYIN)
      | P-(BCIN*D+P)
      | P-(BCIN*D+P+CARRYIN)
      | P-(C)
      | P-(C+(A+D))
      | P-(C+(A+D)*(A+D))
      | P-(C+(A+D)*(A+D)+CARRYIN)
      | P-(C+(A+D)*B)
      | P-(C+(A+D)*B+CARRYIN)
      | P-(C+(A+D)*BCIN)
      | P-(C+(A+D)*BCIN+CARRYIN)
      | P-(C+(A+D)+CARRYIN)
      | P-(C+(ACIN+D))
      | P-(C+(ACIN+D)*(ACIN+D))
      | P-(C+(ACIN+D)*(ACIN+D)+CARRYIN)
      | P-(C+(ACIN+D)*B)
      | P-(C+(ACIN+D)*B+CARRYIN)
      | P-(C+(ACIN+D)*BCIN)
      | P-(C+(ACIN+D)*BCIN+CARRYIN)
      | P-(C+(ACIN+D)+CARRYIN)
      | P-(C+(D+A))
      | P-(C+(D+A)*(D+A))
      | P-(C+(D+A)*(D+A)+CARRYIN)
      | P-(C+(D+A)*B)
      | P-(C+(D+A)*B+CARRYIN)
      | P-(C+(D+A)*BCIN)
      | P-(C+(D+A)*BCIN+CARRYIN)
      | P-(C+(D+A)+CARRYIN)
      | P-(C+(D+ACIN))
      | P-(C+(D+ACIN)*(D+ACIN))
      | P-(C+(D+ACIN)*(D+ACIN)+CARRYIN)
      | P-(C+(D+ACIN)*B)
      | P-(C+(D+ACIN)*B+CARRYIN)
      | P-(C+(D+ACIN)*BCIN)
      | P-(C+(D+ACIN)*BCIN+CARRYIN)
      | P-(C+(D+ACIN)+CARRYIN)
      | P-(C+(D-A))
      | P-(C+(D-A)*(D-A))
      | P-(C+(D-A)*(D-A)+CARRYIN)
      | P-(C+(D-A)*B)
      | P-(C+(D-A)*B+CARRYIN)
      | P-(C+(D-A)*BCIN)
      | P-(C+(D-A)*BCIN+CARRYIN)
      | P-(C+(D-A)+CARRYIN)
      | P-(C+(D-ACIN))
      | P-(C+(D-ACIN)*(D-ACIN))
      | P-(C+(D-ACIN)*(D-ACIN)+CARRYIN)
      | P-(C+(D-ACIN)*B)
      | P-(C+(D-ACIN)*B+CARRYIN)
      | P-(C+(D-ACIN)*BCIN)
      | P-(C+(D-ACIN)*BCIN+CARRYIN)
      | P-(C+(D-ACIN)+CARRYIN)
      | P-(C+A)
      | P-(C+A*A)
      | P-(C+A*A+CARRYIN)
      | P-(C+A*B)
      | P-(C+A*B+CARRYIN)
      | P-(C+A*BCIN)
      | P-(C+A*BCIN+CARRYIN)
      | P-(C+A+CARRYIN)
      | P-(C+ACIN)
      | P-(C+ACIN*ACIN)
      | P-(C+ACIN*ACIN+CARRYIN)
      | P-(C+ACIN*B)
      | P-(C+ACIN*B+CARRYIN)
      | P-(C+ACIN*BCIN)
      | P-(C+ACIN*BCIN+CARRYIN)
      | P-(C+ACIN+CARRYIN)
      | P-(C+B*(A+D))
      | P-(C+B*(A+D)+CARRYIN)
      | P-(C+B*(ACIN+D))
      | P-(C+B*(ACIN+D)+CARRYIN)
      | P-(C+B*(D+A))
      | P-(C+B*(D+A)+CARRYIN)
      | P-(C+B*(D+ACIN))
      | P-(C+B*(D+ACIN)+CARRYIN)
      | P-(C+B*(D-A))
      | P-(C+B*(D-A)+CARRYIN)
      | P-(C+B*(D-ACIN))
      | P-(C+B*(D-ACIN)+CARRYIN)
      | P-(C+B*A)
      | P-(C+B*A+CARRYIN)
      | P-(C+B*ACIN)
      | P-(C+B*ACIN+CARRYIN)
      | P-(C+B*D)
      | P-(C+B*D+CARRYIN)
      | P-(C+BCIN*(A+D))
      | P-(C+BCIN*(A+D)+CARRYIN)
      | P-(C+BCIN*(ACIN+D))
      | P-(C+BCIN*(ACIN+D)+CARRYIN)
      | P-(C+BCIN*(D+A))
      | P-(C+BCIN*(D+A)+CARRYIN)
      | P-(C+BCIN*(D+ACIN))
      | P-(C+BCIN*(D+ACIN)+CARRYIN)
      | P-(C+BCIN*(D-A))
      | P-(C+BCIN*(D-A)+CARRYIN)
      | P-(C+BCIN*(D-ACIN))
      | P-(C+BCIN*(D-ACIN)+CARRYIN)
      | P-(C+BCIN*A)
      | P-(C+BCIN*A+CARRYIN)
      | P-(C+BCIN*ACIN)
      | P-(C+BCIN*ACIN+CARRYIN)
      | P-(C+BCIN*D)
      | P-(C+BCIN*D+CARRYIN)
      | P-(C+C)
      | P-(C+C+CARRYIN)
      | P-(C+C+CONCAT)
      | P-(C+C+CONCAT+CARRYIN)
      | P-(C+C+P)
      | P-(C+C+P+CARRYIN)
      | P-(C+CARRYCASCIN)
      | P-(C+CARRYIN)
      | P-(C+CONCAT)
      | P-(C+CONCAT+C)
      | P-(C+CONCAT+C+CARRYIN)
      | P-(C+CONCAT+CARRYIN)
      | P-(C+CONCAT+P)
      | P-(C+CONCAT+P+CARRYIN)
      | P-(C+D)
      | P-(C+D*B)
      | P-(C+D*B+CARRYIN)
      | P-(C+D*BCIN)
      | P-(C+D*BCIN+CARRYIN)
      | P-(C+D*D)
      | P-(C+D*D+CARRYIN)
      | P-(C+D+CARRYIN)
      | P-(C+P)
      | P-(C+P+C)
      | P-(C+P+C+CARRYIN)
      | P-(C+P+CARRYIN)
      | P-(C+P+CONCAT)
      | P-(C+P+CONCAT+CARRYIN)
      | P-(C+P+P)
      | P-(C+P+P+CARRYIN)
      | P-(CARRYCASCIN)
      | P-(CARRYIN)
      | P-(CONCAT)
      | P-(CONCAT+C)
      | P-(CONCAT+C+C)
      | P-(CONCAT+C+C+CARRYIN)
      | P-(CONCAT+C+CARRYIN)
      | P-(CONCAT+C+P)
      | P-(CONCAT+C+P+CARRYIN)
      | P-(CONCAT+CARRYCASCIN)
      | P-(CONCAT+CARRYIN)
      | P-(CONCAT+P)
      | P-(CONCAT+P+C)
      | P-(CONCAT+P+C+CARRYIN)
      | P-(CONCAT+P+CARRYIN)
      | P-(D)
      | P-(D*B)
      | P-(D*B+C)
      | P-(D*B+C+CARRYIN)
      | P-(D*B+CARRYIN)
      | P-(D*B+P)
      | P-(D*B+P+CARRYIN)
      | P-(D*BCIN)
      | P-(D*BCIN+C)
      | P-(D*BCIN+C+CARRYIN)
      | P-(D*BCIN+CARRYIN)
      | P-(D*BCIN+P)
      | P-(D*BCIN+P+CARRYIN)
      | P-(D*D)
      | P-(D*D+C)
      | P-(D*D+C+CARRYIN)
      | P-(D*D+CARRYIN)
      | P-(D*D+P)
      | P-(D*D+P+CARRYIN)
      | P-(D+A)
      | P-(D+A)*(D+A)
      | P-(D+A)*(D+A)-C
      | P-(D+A)*(D+A)-C-CARRYIN
      | P-(D+A)*(D+A)-CARRYIN
      | P-(D+A)*(D+A)-P
      | P-(D+A)*(D+A)-P-CARRYIN
      | P-(D+A)*B
      | P-(D+A)*B-C
      | P-(D+A)*B-C-CARRYIN
      | P-(D+A)*B-CARRYIN
      | P-(D+A)*B-P
      | P-(D+A)*B-P-CARRYIN
      | P-(D+A)*BCIN
      | P-(D+A)*BCIN-C
      | P-(D+A)*BCIN-C-CARRYIN
      | P-(D+A)*BCIN-CARRYIN
      | P-(D+A)*BCIN-P
      | P-(D+A)*BCIN-P-CARRYIN
      | P-(D+A)-C
      | P-(D+A)-C-CARRYIN
      | P-(D+A)-CARRYIN
      | P-(D+A)-P
      | P-(D+A)-P-CARRYIN
      | P-(D+ACIN)
      | P-(D+ACIN)*(D+ACIN)
      | P-(D+ACIN)*(D+ACIN)-C
      | P-(D+ACIN)*(D+ACIN)-C-CARRYIN
      | P-(D+ACIN)*(D+ACIN)-CARRYIN
      | P-(D+ACIN)*(D+ACIN)-P
      | P-(D+ACIN)*(D+ACIN)-P-CARRYIN
      | P-(D+ACIN)*B
      | P-(D+ACIN)*B-C
      | P-(D+ACIN)*B-C-CARRYIN
      | P-(D+ACIN)*B-CARRYIN
      | P-(D+ACIN)*B-P
      | P-(D+ACIN)*B-P-CARRYIN
      | P-(D+ACIN)*BCIN
      | P-(D+ACIN)*BCIN-C
      | P-(D+ACIN)*BCIN-C-CARRYIN
      | P-(D+ACIN)*BCIN-CARRYIN
      | P-(D+ACIN)*BCIN-P
      | P-(D+ACIN)*BCIN-P-CARRYIN
      | P-(D+ACIN)-C
      | P-(D+ACIN)-C-CARRYIN
      | P-(D+ACIN)-CARRYIN
      | P-(D+ACIN)-P
      | P-(D+ACIN)-P-CARRYIN
      | P-(D+C)
      | P-(D+C+CARRYIN)
      | P-(D+CARRYIN)
      | P-(D+P)
      | P-(D+P+CARRYIN)
      | P-(D-A)
      | P-(D-A)*(D-A)
      | P-(D-A)*(D-A)-C
      | P-(D-A)*(D-A)-C-CARRYIN
      | P-(D-A)*(D-A)-CARRYIN
      | P-(D-A)*(D-A)-P
      | P-(D-A)*(D-A)-P-CARRYIN
      | P-(D-A)*B
      | P-(D-A)*B-C
      | P-(D-A)*B-C-CARRYIN
      | P-(D-A)*B-CARRYIN
      | P-(D-A)*B-P
      | P-(D-A)*B-P-CARRYIN
      | P-(D-A)*BCIN
      | P-(D-A)*BCIN-C
      | P-(D-A)*BCIN-C-CARRYIN
      | P-(D-A)*BCIN-CARRYIN
      | P-(D-A)*BCIN-P
      | P-(D-A)*BCIN-P-CARRYIN
      | P-(D-A)-C
      | P-(D-A)-C-CARRYIN
      | P-(D-A)-CARRYIN
      | P-(D-A)-P
      | P-(D-A)-P-CARRYIN
      | P-(D-ACIN)
      | P-(D-ACIN)*(D-ACIN)
      | P-(D-ACIN)*(D-ACIN)-C
      | P-(D-ACIN)*(D-ACIN)-C-CARRYIN
      | P-(D-ACIN)*(D-ACIN)-CARRYIN
      | P-(D-ACIN)*(D-ACIN)-P
      | P-(D-ACIN)*(D-ACIN)-P-CARRYIN
      | P-(D-ACIN)*B
      | P-(D-ACIN)*B-C
      | P-(D-ACIN)*B-C-CARRYIN
      | P-(D-ACIN)*B-CARRYIN
      | P-(D-ACIN)*B-P
      | P-(D-ACIN)*B-P-CARRYIN
      | P-(D-ACIN)*BCIN
      | P-(D-ACIN)*BCIN-C
      | P-(D-ACIN)*BCIN-C-CARRYIN
      | P-(D-ACIN)*BCIN-CARRYIN
      | P-(D-ACIN)*BCIN-P
      | P-(D-ACIN)*BCIN-P-CARRYIN
      | P-(D-ACIN)-C
      | P-(D-ACIN)-C-CARRYIN
      | P-(D-ACIN)-CARRYIN
      | P-(D-ACIN)-P
      | P-(D-ACIN)-P-CARRYIN
      | P-(P)
      | P-(P+(A+D))
      | P-(P+(A+D)*(A+D))
      | P-(P+(A+D)*(A+D)+CARRYIN)
      | P-(P+(A+D)*B)
      | P-(P+(A+D)*B+CARRYIN)
      | P-(P+(A+D)*BCIN)
      | P-(P+(A+D)*BCIN+CARRYIN)
      | P-(P+(A+D)+CARRYIN)
      | P-(P+(ACIN+D))
      | P-(P+(ACIN+D)*(ACIN+D))
      | P-(P+(ACIN+D)*(ACIN+D)+CARRYIN)
      | P-(P+(ACIN+D)*B)
      | P-(P+(ACIN+D)*B+CARRYIN)
      | P-(P+(ACIN+D)*BCIN)
      | P-(P+(ACIN+D)*BCIN+CARRYIN)
      | P-(P+(ACIN+D)+CARRYIN)
      | P-(P+(D+A))
      | P-(P+(D+A)*(D+A))
      | P-(P+(D+A)*(D+A)+CARRYIN)
      | P-(P+(D+A)*B)
      | P-(P+(D+A)*B+CARRYIN)
      | P-(P+(D+A)*BCIN)
      | P-(P+(D+A)*BCIN+CARRYIN)
      | P-(P+(D+A)+CARRYIN)
      | P-(P+(D+ACIN))
      | P-(P+(D+ACIN)*(D+ACIN))
      | P-(P+(D+ACIN)*(D+ACIN)+CARRYIN)
      | P-(P+(D+ACIN)*B)
      | P-(P+(D+ACIN)*B+CARRYIN)
      | P-(P+(D+ACIN)*BCIN)
      | P-(P+(D+ACIN)*BCIN+CARRYIN)
      | P-(P+(D+ACIN)+CARRYIN)
      | P-(P+(D-A))
      | P-(P+(D-A)*(D-A))
      | P-(P+(D-A)*(D-A)+CARRYIN)
      | P-(P+(D-A)*B)
      | P-(P+(D-A)*B+CARRYIN)
      | P-(P+(D-A)*BCIN)
      | P-(P+(D-A)*BCIN+CARRYIN)
      | P-(P+(D-A)+CARRYIN)
      | P-(P+(D-ACIN))
      | P-(P+(D-ACIN)*(D-ACIN))
      | P-(P+(D-ACIN)*(D-ACIN)+CARRYIN)
      | P-(P+(D-ACIN)*B)
      | P-(P+(D-ACIN)*B+CARRYIN)
      | P-(P+(D-ACIN)*BCIN)
      | P-(P+(D-ACIN)*BCIN+CARRYIN)
      | P-(P+(D-ACIN)+CARRYIN)
      | P-(P+A)
      | P-(P+A*A)
      | P-(P+A*A+CARRYIN)
      | P-(P+A*B)
      | P-(P+A*B+CARRYIN)
      | P-(P+A*BCIN)
      | P-(P+A*BCIN+CARRYIN)
      | P-(P+A+CARRYIN)
      | P-(P+ACIN)
      | P-(P+ACIN*ACIN)
      | P-(P+ACIN*ACIN+CARRYIN)
      | P-(P+ACIN*B)
      | P-(P+ACIN*B+CARRYIN)
      | P-(P+ACIN*BCIN)
      | P-(P+ACIN*BCIN+CARRYIN)
      | P-(P+ACIN+CARRYIN)
      | P-(P+B*(A+D))
      | P-(P+B*(A+D)+CARRYIN)
      | P-(P+B*(ACIN+D))
      | P-(P+B*(ACIN+D)+CARRYIN)
      | P-(P+B*(D+A))
      | P-(P+B*(D+A)+CARRYIN)
      | P-(P+B*(D+ACIN))
      | P-(P+B*(D+ACIN)+CARRYIN)
      | P-(P+B*(D-A))
      | P-(P+B*(D-A)+CARRYIN)
      | P-(P+B*(D-ACIN))
      | P-(P+B*(D-ACIN)+CARRYIN)
      | P-(P+B*A)
      | P-(P+B*A+CARRYIN)
      | P-(P+B*ACIN)
      | P-(P+B*ACIN+CARRYIN)
      | P-(P+B*D)
      | P-(P+B*D+CARRYIN)
      | P-(P+BCIN*(A+D))
      | P-(P+BCIN*(A+D)+CARRYIN)
      | P-(P+BCIN*(ACIN+D))
      | P-(P+BCIN*(ACIN+D)+CARRYIN)
      | P-(P+BCIN*(D+A))
      | P-(P+BCIN*(D+A)+CARRYIN)
      | P-(P+BCIN*(D+ACIN))
      | P-(P+BCIN*(D+ACIN)+CARRYIN)
      | P-(P+BCIN*(D-A))
      | P-(P+BCIN*(D-A)+CARRYIN)
      | P-(P+BCIN*(D-ACIN))
      | P-(P+BCIN*(D-ACIN)+CARRYIN)
      | P-(P+BCIN*A)
      | P-(P+BCIN*A+CARRYIN)
      | P-(P+BCIN*ACIN)
      | P-(P+BCIN*ACIN+CARRYIN)
      | P-(P+BCIN*D)